simulink model "MX_Gateway"  (Simulink 9.0)
settings: Solver=FixedStepDiscrete  StartTime=0.0  StopTime=100  FixedStep=0.01  RelTol=1e-3
values from neighbouring files (symbols this model uses):
  ACTIVE = Bobcat.Parameter; CreateDefine( ACTIVE                         ,1                          ,'Define' ,'defines.h' )    [defines.m]
  ACTIVE_MODE_ONE = Bobcat.Parameter; CreateDefine( ACTIVE_MODE_ONE                ,19                         ,'Define' ,'defines.h' )    [defines.m]
  AUTHENTICATED = Bobcat.Parameter; CreateDefine( AUTHENTICATED                  ,1                          ,'Define' ,'defines.h' )    [defines.m]
  AUX_EMEA_CONTROLS = Bobcat.Parameter; CreateDefine( AUX_EMEA_CONTROLS  ,1   ,'Define' ,'defines.h' )    [enums.m]
  AUX_FLOW_HIGH = Bobcat.Parameter; CreateDefine( AUX_FLOW_HIGH           ,4  ,'Define' ,'defines.h' )    [enums.m]
  AUX_FLOW_INACTIVE = Bobcat.Parameter; CreateDefine( AUX_FLOW_INACTIVE       ,0  ,'Define' ,'defines.h' )    [enums.m]
  AUX_FLOW_LOW = Bobcat.Parameter; CreateDefine( AUX_FLOW_LOW            ,1  ,'Define' ,'defines.h' )    [enums.m]
  AUX_FLOW_MEDIUM = Bobcat.Parameter; CreateDefine( AUX_FLOW_MEDIUM         ,2  ,'Define' ,'defines.h' )    [enums.m]
  AUX_FLOW_STANDARD = Bobcat.Parameter; CreateDefine( AUX_FLOW_STANDARD       ,3  ,'Define' ,'defines.h' )    [enums.m]
  AUX_NON_FUNCTIONAL = Bobcat.Parameter; CreateDefine( AUX_NON_FUNCTIONAL ,255 ,'Define' ,'defines.h' )    [enums.m]
  BASE_ACTIVE = Bobcat.Parameter; CreateDefine( BASE_ACTIVE                    ,253                        ,'Define' ,'defines.h' )    [defines.m]
  BASE_DETENT = Bobcat.Parameter; CreateDefine( BASE_DETENT                    ,1                          ,'Define' ,'defines.h' )    [defines.m]
  BEEP_NONE = Bobcat.Parameter; CreateDefine( BEEP_NONE                      ,255                        ,'Define' ,'defines.h' )    [defines.m]
  CD_AIR_FILTER_DEBOUNCE_NORMAL = Bobcat.Parameter; CreateAliasCD( CD_AIR_FILTER_DEBOUNCE_NORMAL                        ,uint16(500)                ,'ConstantDataPointer' ,'CONSTANT_DATA' ,'AIR_FILTER_DEBOUNCE_NORMAL')    [cdata.m]    (also in hc12_cdata.m; m4_cdata.m)
  CD_AIR_FILTER_DEBOUNCE_NOT_CONNECTED = Bobcat.Parameter; CreateAliasCD( CD_AIR_FILTER_DEBOUNCE_NOT_CONNECTED                 ,uint16(3000)               ,'ConstantDataPointer' ,'CONSTANT_DATA' ,'AIR_FILTER_DEBOUNCE_NOT_CONNECTED')    [cdata.m]    (also in hc12_cdata.m; m4_cdata.m)
  CD_AIR_FILTER_DEBOUNCE_PLUGGED = Bobcat.Parameter; CreateAliasCD( CD_AIR_FILTER_DEBOUNCE_PLUGGED                       ,uint16(3000)               ,'ConstantDataPointer' ,'CONSTANT_DATA' ,'AIR_FILTER_DEBOUNCE_PLUGGED')    [cdata.m]    (also in hc12_cdata.m; m4_cdata.m)
  CD_AIR_FILTER_FUNCTION = Bobcat.Parameter; CreateAliasCD( CD_AIR_FILTER_FUNCTION                               ,uint8(255)                 ,'ConstantDataPointer' ,'CONSTANT_DATA' ,'AIR_FILTER_FUNCTION')    [cdata.m]    (also in hc12_cdata.m; m4_cdata.m)
  CD_AIR_FILTER_PLUGGED_WEIGHT = Bobcat.Parameter; CreateAliasCD( CD_AIR_FILTER_PLUGGED_WEIGHT                         ,uint8(2)                   ,'ConstantDataPointer' ,'CONSTANT_DATA' ,'AIR_FILTER_PLUGGED_WEIGHT')    [cdata.m]    (also in hc12_cdata.m; m4_cdata.m)
  CD_AIR_FILTER_UNPLUGGED_WEIGHT = Bobcat.Parameter; CreateAliasCD( CD_AIR_FILTER_UNPLUGGED_WEIGHT                       ,uint8(1)                   ,'ConstantDataPointer' ,'CONSTANT_DATA' ,'AIR_FILTER_UNPLUGGED_WEIGHT')    [cdata.m]    (also in hc12_cdata.m; m4_cdata.m)
  CD_AUTOIDLE_ARMBAR_DELAY = Bobcat.Parameter; CreateAliasCD( CD_AUTOIDLE_ARMBAR_DELAY                             ,uint8(2)                   ,'ConstantDataPointer' ,'CONSTANT_DATA' ,'AUTOIDLE_ARMBAR_DELAY')    [cdata.m]    (also in hc12_cdata.m; m4_cdata.m)
  CD_AUTOIDLE_FUNCTION = Bobcat.Parameter; CreateAliasCD( CD_AUTOIDLE_FUNCTION                                 ,uint8(255)                 ,'ConstantDataPointer' ,'CONSTANT_DATA' ,'AUTOIDLE_FUNCTION')    [cdata.m]    (also in hc12_cdata.m; m4_cdata.m)
  CD_AUTOIDLE_IDLE_DELAY = Bobcat.Parameter; CreateAliasCD( CD_AUTOIDLE_IDLE_DELAY                               ,uint8(40)                  ,'ConstantDataPointer' ,'CONSTANT_DATA' ,'AUTOIDLE_IDLE_DELAY')    [cdata.m]    (also in hc12_cdata.m; m4_cdata.m)
  CD_AUTOIDLE_LOW_IDLE_START = Bobcat.Parameter; CreateAliasCD( CD_AUTOIDLE_LOW_IDLE_START                           ,uint8(255)                 ,'ConstantDataPointer' ,'CONSTANT_DATA' ,'AUTOIDLE_LOW_IDLE_START')    [cdata.m]    (also in hc12_cdata.m; m4_cdata.m)
  CD_AUTOIDLE_PRESSURE_OFF = Bobcat.Parameter; CreateAliasCD( CD_AUTOIDLE_PRESSURE_OFF                             ,uint16(300)                ,'ConstantDataPointer' ,'CONSTANT_DATA' ,'AUTOIDLE_PRESSURE_OFF')    [cdata.m]    (also in hc12_cdata.m; m4_cdata.m)
  CD_AUTOIDLE_PRESSURE_ON = Bobcat.Parameter; CreateAliasCD( CD_AUTOIDLE_PRESSURE_ON                              ,uint16(270)                ,'ConstantDataPointer' ,'CONSTANT_DATA' ,'AUTOIDLE_PRESSURE_ON')    [cdata.m]    (also in hc12_cdata.m; m4_cdata.m)
  CD_AUTOSHIFT_FUNCTION = Bobcat.Parameter; CreateAliasCD( CD_AUTOSHIFT_FUNCTION                                ,uint8(255)                 ,'ConstantDataPointer' ,'CONSTANT_DATA' ,'AUTOSHIFT_FUNCTION')    [cdata.m]    (also in hc12_cdata.m; m4_cdata.m)
  CD_AUTOSHIFT_PHIGH1 = Bobcat.Parameter; CreateAliasCD( CD_AUTOSHIFT_PHIGH1                                  ,uint16(205)                ,'ConstantDataPointer' ,'CONSTANT_DATA' ,'AUTOSHIFT_PHIGH1')    [cdata.m]    (also in hc12_cdata.m; m4_cdata.m)
  CD_AUTOSHIFT_PHIGH2 = Bobcat.Parameter; CreateAliasCD( CD_AUTOSHIFT_PHIGH2                                  ,uint16(193)                ,'ConstantDataPointer' ,'CONSTANT_DATA' ,'AUTOSHIFT_PHIGH2')    [cdata.m]    (also in hc12_cdata.m; m4_cdata.m)
  CD_AUTOSHIFT_PHIGH_TEMP1 = Bobcat.Parameter; CreateAliasCD( CD_AUTOSHIFT_PHIGH_TEMP1                             ,int16(70)                  ,'ConstantDataPointer' ,'CONSTANT_DATA' ,'AUTOSHIFT_PHIGH_TEMP1')    [cdata.m]    (also in hc12_cdata.m; m4_cdata.m)
  CD_AUTOSHIFT_PHIGH_TEMP2 = Bobcat.Parameter; CreateAliasCD( CD_AUTOSHIFT_PHIGH_TEMP2                             ,int16(160)                 ,'ConstantDataPointer' ,'CONSTANT_DATA' ,'AUTOSHIFT_PHIGH_TEMP2')    [cdata.m]    (also in hc12_cdata.m; m4_cdata.m)
  CD_AUTOSHIFT_PLOW1 = Bobcat.Parameter; CreateAliasCD( CD_AUTOSHIFT_PLOW1                                   ,uint16(130)                ,'ConstantDataPointer' ,'CONSTANT_DATA' ,'AUTOSHIFT_PLOW1')    [cdata.m]    (also in hc12_cdata.m; m4_cdata.m)
  CD_AUTOSHIFT_PLOW2 = Bobcat.Parameter; CreateAliasCD( CD_AUTOSHIFT_PLOW2                                   ,uint16(100)                ,'ConstantDataPointer' ,'CONSTANT_DATA' ,'AUTOSHIFT_PLOW2')    [cdata.m]    (also in hc12_cdata.m; m4_cdata.m)
  CD_AUTOSHIFT_PLOW_TEMP1 = Bobcat.Parameter; CreateAliasCD( CD_AUTOSHIFT_PLOW_TEMP1                              ,int16(20)                  ,'ConstantDataPointer' ,'CONSTANT_DATA' ,'AUTOSHIFT_PLOW_TEMP1')    [cdata.m]    (also in hc12_cdata.m; m4_cdata.m)
  CD_AUTOSHIFT_PLOW_TEMP2 = Bobcat.Parameter; CreateAliasCD( CD_AUTOSHIFT_PLOW_TEMP2                              ,int16(80)                  ,'ConstantDataPointer' ,'CONSTANT_DATA' ,'AUTOSHIFT_PLOW_TEMP2')    [cdata.m]    (also in hc12_cdata.m; m4_cdata.m)
  CD_AUTOSHIFT_TRAVEL_DELAY = Bobcat.Parameter; CreateAliasCD( CD_AUTOSHIFT_TRAVEL_DELAY                            ,uint8(32)                  ,'ConstantDataPointer' ,'CONSTANT_DATA' ,'AUTOSHIFT_TRAVEL_DELAY')    [cdata.m]    (also in hc12_cdata.m; m4_cdata.m)
  CD_AUX2_CRACKPOINT_CURRENT = Bobcat.Parameter; CreateAliasCD( CD_AUX2_CRACKPOINT_CURRENT                           ,fi(0,0,16,12,'hex','0c28') ,'ConstantDataPointer' ,'CONSTANT_DATA' ,'AUX2_CRACKPOINT_CURRENT')    [cdata.m]    (also in hc12_cdata.m; m4_cdata.m)
  CD_AUX2_FLOW_LOW_FUNCTION = Bobcat.Parameter; CreateAliasCD( CD_AUX2_FLOW_LOW_FUNCTION                            ,uint8(255)                 ,'ConstantDataPointer' ,'CONSTANT_DATA' ,'AUX2_FLOW_LOW_FUNCTION')    [cdata.m]    (also in hc12_cdata.m; m4_cdata.m)
  CD_AUX2_FLOW_MED_FUNCTION = Bobcat.Parameter; CreateAliasCD( CD_AUX2_FLOW_MED_FUNCTION                            ,uint8(255)                 ,'ConstantDataPointer' ,'CONSTANT_DATA' ,'AUX2_FLOW_MED_FUNCTION')    [cdata.m]    (also in hc12_cdata.m; m4_cdata.m)
  CD_AUX2_HI_FLOW_PERCENT = Bobcat.Parameter; CreateAliasCD( CD_AUX2_HI_FLOW_PERCENT                              ,fi(0,0,16,16,'hex','ffff') ,'ConstantDataPointer' ,'CONSTANT_DATA' ,'AUX2_HI_FLOW_PERCENT')    [cdata.m]    (also in hc12_cdata.m; m4_cdata.m)
  CD_AUX2_LOW_FLOW_PERCENT = Bobcat.Parameter; CreateAliasCD( CD_AUX2_LOW_FLOW_PERCENT                             ,fi(0,0,16,16,'hex','7333') ,'ConstantDataPointer' ,'CONSTANT_DATA' ,'AUX2_LOW_FLOW_PERCENT')    [cdata.m]    (also in hc12_cdata.m; m4_cdata.m)
  CD_AUX2_MAX_FLOW_CURRENT = Bobcat.Parameter; CreateAliasCD( CD_AUX2_MAX_FLOW_CURRENT                             ,fi(0,0,16,12,'hex','14cc') ,'ConstantDataPointer' ,'CONSTANT_DATA' ,'AUX2_MAX_FLOW_CURRENT')    [cdata.m]    (also in hc12_cdata.m; m4_cdata.m)
  CD_AUX2_MED_FLOW_PERCENT = Bobcat.Parameter; CreateAliasCD( CD_AUX2_MED_FLOW_PERCENT                             ,fi(0,0,16,16,'hex','9999') ,'ConstantDataPointer' ,'CONSTANT_DATA' ,'AUX2_MED_FLOW_PERCENT')    [cdata.m]    (also in hc12_cdata.m; m4_cdata.m)
  CD_AUX_CRACKPOINT_CURRENT = Bobcat.Parameter; CreateAliasCD( CD_AUX_CRACKPOINT_CURRENT                            ,fi(0,0,16,12,'hex','0000') ,'ConstantDataPointer' ,'CONSTANT_DATA' ,'AUX_CRACKPOINT_CURRENT')    [cdata.m]    (also in hc12_cdata.m; m4_cdata.m)
  CD_AUX_DETENT_FLASH_TIME = Bobcat.Parameter; CreateAliasCD( CD_AUX_DETENT_FLASH_TIME                             ,uint16(72)                 ,'ConstantDataPointer' ,'CONSTANT_DATA' ,'AUX_DETENT_FLASH_TIME')    [cdata.m]    (also in hc12_cdata.m; m4_cdata.m)
  CD_AUX_DETENT_HOLD_TIME = Bobcat.Parameter; CreateAliasCD( CD_AUX_DETENT_HOLD_TIME                              ,uint16(100)                ,'ConstantDataPointer' ,'CONSTANT_DATA' ,'AUX_DETENT_HOLD_TIME')    [cdata.m]    (also in hc12_cdata.m; m4_cdata.m)
  CD_AUX_FULL_ON_AUX1 = Bobcat.Parameter; CreateAliasCD( CD_AUX_FULL_ON_AUX1                                  ,fi(0,0,16,12,'hex','10cd') ,'ConstantDataPointer' ,'CONSTANT_DATA' ,'AUX_FULL_ON_AUX1')    [cdata.m]    (also in hc12_cdata.m; m4_cdata.m)
  CD_AUX_FULL_ON_AUX2 = Bobcat.Parameter; CreateAliasCD( CD_AUX_FULL_ON_AUX2                                  ,fi(0,0,16,12,'hex','139d') ,'ConstantDataPointer' ,'CONSTANT_DATA' ,'AUX_FULL_ON_AUX2')    [cdata.m]    (also in hc12_cdata.m; m4_cdata.m)
  CD_AUX_FULL_ON_AUX3 = Bobcat.Parameter; CreateAliasCD( CD_AUX_FULL_ON_AUX3                                  ,fi(0,0,16,12,'hex','17d7') ,'ConstantDataPointer' ,'CONSTANT_DATA' ,'AUX_FULL_ON_AUX3')    [cdata.m]    (also in hc12_cdata.m; m4_cdata.m)
  CD_AUX_FUNCTION = Bobcat.Parameter; CreateAliasCD( CD_AUX_FUNCTION                                      ,uint8(0)                   ,'ConstantDataPointer' ,'CONSTANT_DATA' ,'AUX_FUNCTION')    [cdata.m]    (also in hc12_cdata.m; m4_cdata.m)
  CD_AUX_RATE_LIMIT_CRACKPOINT = Bobcat.Parameter; CreateAliasCD( CD_AUX_RATE_LIMIT_CRACKPOINT                         ,fi(0,0,16,12,'hex','0800') ,'ConstantDataPointer' ,'CONSTANT_DATA' ,'AUX_RATE_LIMIT_CRACKPOINT')    [cdata.m]    (also in hc12_cdata.m; m4_cdata.m)
  CD_AUX_RATE_LIMIT_CRACKPOINT_RATE = Bobcat.Parameter; CreateAliasCD( CD_AUX_RATE_LIMIT_CRACKPOINT_RATE                    ,fi(0,1,32,12,'int',102400) ,'ConstantDataPointer' ,'CONSTANT_DATA' ,'AUX_RATE_LIMIT_CRACKPOINT_RATE')    [cdata.m]    (also in hc12_cdata.m; m4_cdata.m)
  CD_AUX_RATE_LIMIT_FUNCTION = Bobcat.Parameter; CreateAliasCD( CD_AUX_RATE_LIMIT_FUNCTION                           ,uint8(255)                 ,'ConstantDataPointer' ,'CONSTANT_DATA' ,'AUX_RATE_LIMIT_FUNCTION')    [cdata.m]    (also in hc12_cdata.m; m4_cdata.m)
  CD_AUX_RATE_LIMIT_MAX_ENG_SPD = Bobcat.Parameter; CreateAliasCD( CD_AUX_RATE_LIMIT_MAX_ENG_SPD                        ,uint16(1600)               ,'ConstantDataPointer' ,'CONSTANT_DATA' ,'AUX_RATE_LIMIT_MAX_ENG_SPD')    [cdata.m]    (also in hc12_cdata.m; m4_cdata.m)
  CD_AUX_RATE_LIMIT_MIN_ENG_SPD = Bobcat.Parameter; CreateAliasCD( CD_AUX_RATE_LIMIT_MIN_ENG_SPD                        ,uint16(100)                ,'ConstantDataPointer' ,'CONSTANT_DATA' ,'AUX_RATE_LIMIT_MIN_ENG_SPD')    [cdata.m]    (also in hc12_cdata.m; m4_cdata.m)
  CD_AUX_RATE_LIMIT_NORMAL_RATE = Bobcat.Parameter; CreateAliasCD( CD_AUX_RATE_LIMIT_NORMAL_RATE                        ,fi(0,1,32,12,'int',2048)   ,'ConstantDataPointer' ,'CONSTANT_DATA' ,'AUX_RATE_LIMIT_NORMAL_RATE')    [cdata.m]    (also in hc12_cdata.m; m4_cdata.m)
  CD_AUXPOT_CAL_DEADBAND_HIGH = Bobcat.Parameter; CreateAliasCD( CD_AUXPOT_CAL_DEADBAND_HIGH                          ,uint16(574)                ,'ConstantDataPointer' ,'CONSTANT_DATA' ,'AUXPOT_CAL_DEADBAND_HIGH')    [cdata.m]    (also in hc12_cdata.m; m4_cdata.m)
  CD_AUXPOT_CAL_DEADBAND_LOW = Bobcat.Parameter; CreateAliasCD( CD_AUXPOT_CAL_DEADBAND_LOW                           ,uint16(424)                ,'ConstantDataPointer' ,'CONSTANT_DATA' ,'AUXPOT_CAL_DEADBAND_LOW')    [cdata.m]    (also in hc12_cdata.m; m4_cdata.m)
  CD_AUXPOT_CENTER_RATIO = Bobcat.Parameter; CreateAliasCD( CD_AUXPOT_CENTER_RATIO                               ,uint16(500)                ,'ConstantDataPointer' ,'CONSTANT_DATA' ,'AUXPOT_CENTER_RATIO')    [cdata.m]    (also in hc12_cdata.m; m4_cdata.m)
  CD_AUXPOT_DEADBAND = Bobcat.Parameter; CreateAliasCD( CD_AUXPOT_DEADBAND                                   ,uint8(38)                  ,'ConstantDataPointer' ,'CONSTANT_DATA' ,'AUXPOT_DEADBAND')    [cdata.m]    (also in hc12_cdata.m; m4_cdata.m)
  CD_AUXPOT_FUNCTION = Bobcat.Parameter; CreateAliasCD( CD_AUXPOT_FUNCTION                                   ,uint8(0)                   ,'ConstantDataPointer' ,'CONSTANT_DATA' ,'AUXPOT_FUNCTION')    [cdata.m]    (also in hc12_cdata.m; m4_cdata.m)
  CD_AUXPOT_RATIO_GAIN = Bobcat.Parameter; CreateAliasCD( CD_AUXPOT_RATIO_GAIN                                 ,uint16(1000)               ,'ConstantDataPointer' ,'CONSTANT_DATA' ,'AUXPOT_RATIO_GAIN')    [cdata.m]    (also in hc12_cdata.m; m4_cdata.m)
  CD_CAN_THROTTLE_FUNCTION = Bobcat.Parameter; CreateAliasCD( CD_CAN_THROTTLE_FUNCTION                             ,uint8(255)                 ,'ConstantDataPointer' ,'CONSTANT_DATA' ,'CAN_THROTTLE_FUNCTION')    [cdata.m]    (also in hc12_cdata.m; m4_cdata.m)
  CD_CAN_THROTTLE_REVERSE = Bobcat.Parameter; CreateAliasCD( CD_CAN_THROTTLE_REVERSE                              ,uint8(255)                 ,'ConstantDataPointer' ,'CONSTANT_DATA' ,'CAN_THROTTLE_REVERSE')    [cdata.m]    (also in hc12_cdata.m; m4_cdata.m)
  CD_CONSOLE_DEBOUNCE_ABSENT = Bobcat.Parameter; CreateAliasCD( CD_CONSOLE_DEBOUNCE_ABSENT                           ,uint16(12)                 ,'ConstantDataPointer' ,'CONSTANT_DATA' ,'CONSOLE_DEBOUNCE_ABSENT')    [cdata.m]    (also in hc12_cdata.m; m4_cdata.m)
  CD_CONSOLE_DEBOUNCE_OORH = Bobcat.Parameter; CreateAliasCD( CD_CONSOLE_DEBOUNCE_OORH                             ,uint16(12)                 ,'ConstantDataPointer' ,'CONSTANT_DATA' ,'CONSOLE_DEBOUNCE_OORH')    [cdata.m]    (also in hc12_cdata.m; m4_cdata.m)
  CD_CONSOLE_DEBOUNCE_OORL = Bobcat.Parameter; CreateAliasCD( CD_CONSOLE_DEBOUNCE_OORL                             ,uint16(12)                 ,'ConstantDataPointer' ,'CONSTANT_DATA' ,'CONSOLE_DEBOUNCE_OORL')    [cdata.m]    (also in hc12_cdata.m; m4_cdata.m)
  CD_CONSOLE_DEBOUNCE_PRESENT = Bobcat.Parameter; CreateAliasCD( CD_CONSOLE_DEBOUNCE_PRESENT                          ,uint16(12)                 ,'ConstantDataPointer' ,'CONSTANT_DATA' ,'CONSOLE_DEBOUNCE_PRESENT')    [cdata.m]    (also in hc12_cdata.m; m4_cdata.m)
  CD_CONSOLE_FUNCTION = Bobcat.Parameter; CreateAliasCD( CD_CONSOLE_FUNCTION                                  ,uint8(0)                   ,'ConstantDataPointer' ,'CONSTANT_DATA' ,'CONSOLE_FUNCTION')    [cdata.m]    (also in hc12_cdata.m; m4_cdata.m)
  CD_CONSOLE_OORH = Bobcat.Parameter; CreateAliasCD( CD_CONSOLE_OORH                                      ,uint16(740)                ,'ConstantDataPointer' ,'CONSTANT_DATA' ,'CONSOLE_OORH')    [cdata.m]    (also in hc12_cdata.m; m4_cdata.m)
  CD_CONSOLE_OORL = Bobcat.Parameter; CreateAliasCD( CD_CONSOLE_OORL                                      ,uint16(10)                 ,'ConstantDataPointer' ,'CONSTANT_DATA' ,'CONSOLE_OORL')    [cdata.m]    (also in hc12_cdata.m; m4_cdata.m)
  CD_CONSOLE_PRESENT_HIGH = Bobcat.Parameter; CreateAliasCD( CD_CONSOLE_PRESENT_HIGH                              ,uint16(515)                ,'ConstantDataPointer' ,'CONSTANT_DATA' ,'CONSOLE_PRESENT_HIGH')    [cdata.m]    (also in hc12_cdata.m; m4_cdata.m)
  CD_CONSOLE_PRESENT_LOW = Bobcat.Parameter; CreateAliasCD( CD_CONSOLE_PRESENT_LOW                               ,uint16(375)                ,'ConstantDataPointer' ,'CONSTANT_DATA' ,'CONSOLE_PRESENT_LOW')    [cdata.m]    (also in hc12_cdata.m; m4_cdata.m)
  CD_DELUXE_GEN5_5_FUNCTION = Bobcat.Parameter; CreateAliasCD( CD_DELUXE_GEN5_5_FUNCTION                            ,uint8(255)                 ,'ConstantDataPointer' ,'CONSTANT_DATA' ,'DELUXE_GEN5_5_FUNCTION')    [cdata.m]    (also in hc12_cdata.m; m4_cdata.m)
  CD_DELUXE_GEN5_FUNCTION = Bobcat.Parameter; CreateAliasCD( CD_DELUXE_GEN5_FUNCTION                              ,uint8(255)                 ,'ConstantDataPointer' ,'CONSTANT_DATA' ,'DELUXE_GEN5_FUNCTION')    [cdata.m]    (also in hc12_cdata.m; m4_cdata.m)
  CD_DERATE_ENGINE_SPEED_DEBOUNCE_HIGH = Bobcat.Parameter; CreateAliasCD( CD_DERATE_ENGINE_SPEED_DEBOUNCE_HIGH                 ,uint16(200)                ,'ConstantDataPointer' ,'CONSTANT_DATA' ,'DERATE_ENGINE_SPEED_DEBOUNCE_HIGH')    [cdata.m]    (also in hc12_cdata.m; m4_cdata.m)
  CD_DERATE_ENGINE_SPEED_DEBOUNCE_SHUTDOWN = Bobcat.Parameter; CreateAliasCD( CD_DERATE_ENGINE_SPEED_DEBOUNCE_SHUTDOWN             ,uint16(1000)               ,'ConstantDataPointer' ,'CONSTANT_DATA' ,'DERATE_ENGINE_SPEED_DEBOUNCE_SHUTDOWN')    [cdata.m]    (also in hc12_cdata.m; m4_cdata.m)
  CD_DERATE_ENGINE_SPEED_HIGH_OFFSET = Bobcat.Parameter; CreateAliasCD( CD_DERATE_ENGINE_SPEED_HIGH_OFFSET                   ,uint16(200)                ,'ConstantDataPointer' ,'CONSTANT_DATA' ,'DERATE_ENGINE_SPEED_HIGH_OFFSET')    [cdata.m]    (also in hc12_cdata.m; m4_cdata.m)
  CD_DERATE_METHOD = Bobcat.Parameter; CreateAliasCD( CD_DERATE_METHOD                                     ,uint8(0)                   ,'ConstantDataPointer' ,'CONSTANT_DATA' ,'DERATE_METHOD')    [cdata.m]    (also in hc12_cdata.m; m4_cdata.m)
  CD_DUMP_VALVE_FUNCTION = Bobcat.Parameter; CreateAliasCD( CD_DUMP_VALVE_FUNCTION                               ,uint8(255)                 ,'ConstantDataPointer' ,'CONSTANT_DATA' ,'DUMP_VALVE_FUNCTION')    [cdata.m]    (also in hc12_cdata.m; m4_cdata.m)
  CD_DUMP_VALVE_ON_CURRENT = Bobcat.Parameter; CreateAliasCD( CD_DUMP_VALVE_ON_CURRENT                             ,fi(0,0,16,12,'hex','ffff') ,'ConstantDataPointer' ,'CONSTANT_DATA' ,'DUMP_VALVE_ON_CURRENT')    [cdata.m]    (also in hc12_cdata.m; m4_cdata.m)
  CD_ECO_MODE_THROTTLE_REDUCTION = Bobcat.Parameter; CreateAliasCD( CD_ECO_MODE_THROTTLE_REDUCTION                       ,uint16(850)                ,'ConstantDataPointer' ,'CONSTANT_DATA' ,'ECO_MODE_THROTTLE_REDUCTION')    [cdata.m]    (also in hc12_cdata.m; m4_cdata.m)
  CD_ECT_FILTER_GAIN = Bobcat.Parameter; CreateAliasCD( CD_ECT_FILTER_GAIN                                   ,uint8(6)                   ,'ConstantDataPointer' ,'CONSTANT_DATA' ,'ECT_FILTER_GAIN')    [cdata.m]    (also in hc12_cdata.m; m4_cdata.m)
  CD_ECU_FUNCTION = Bobcat.Parameter; CreateAliasCD( CD_ECU_FUNCTION                                      ,uint8(255)                 ,'ConstantDataPointer' ,'CONSTANT_DATA' ,'ECU_FUNCTION')    [cdata.m]    (also in hc12_cdata.m; m4_cdata.m)
  CD_ECU_HOLD_DEBOUNCE_ERROR_ON = Bobcat.Parameter; CreateAliasCD( CD_ECU_HOLD_DEBOUNCE_ERROR_ON                        ,uint16(20)                 ,'ConstantDataPointer' ,'CONSTANT_DATA' ,'ECU_HOLD_DEBOUNCE_ERROR_ON')    [cdata.m]    (also in hc12_cdata.m; m4_cdata.m)
  CD_EMEA_CONTROLS_HOLD_TIME = Bobcat.Parameter; CreateAliasCD( CD_EMEA_CONTROLS_HOLD_TIME                           ,uint16(30)                 ,'ConstantDataPointer' ,'CONSTANT_DATA' ,'EMEA_CONTROLS_HOLD_TIME')    [cdata.m]    (also in hc12_cdata.m; m4_cdata.m)
  CD_EMEA_CONTROLS_OPTION_A = Bobcat.Parameter; CreateAliasCD( CD_EMEA_CONTROLS_OPTION_A                            ,uint8(255)                 ,'ConstantDataPointer' ,'CONSTANT_DATA' ,'EMEA_CONTROLS_OPTION_A')    [cdata.m]    (also in hc12_cdata.m; m4_cdata.m)
  CD_EMEA_CONTROLS_OPTION_C = Bobcat.Parameter; CreateAliasCD( CD_EMEA_CONTROLS_OPTION_C                            ,uint8(255)                 ,'ConstantDataPointer' ,'CONSTANT_DATA' ,'EMEA_CONTROLS_OPTION_C')    [cdata.m]    (also in hc12_cdata.m; m4_cdata.m)
  CD_EMEA_CONTROLS_REVERSE = Bobcat.Parameter; CreateAliasCD( CD_EMEA_CONTROLS_REVERSE                             ,uint8(255)                 ,'ConstantDataPointer' ,'CONSTANT_DATA' ,'EMEA_CONTROLS_REVERSE')    [cdata.m]    (also in hc12_cdata.m; m4_cdata.m)
  CD_EMEA_ELECTRIC_VERSION = Bobcat.Parameter; CreateAliasCD( CD_EMEA_ELECTRIC_VERSION                             ,uint8(0)                   ,'ConstantDataPointer' ,'CONSTANT_DATA' ,'EMEA_ELECTRIC_VERSION')    [cdata.m]    (also in hc12_cdata.m; m4_cdata.m)
  CD_EMEA_POT_CAL_DEADBAND_HIGH = Bobcat.Parameter; CreateAliasCD( CD_EMEA_POT_CAL_DEADBAND_HIGH                        ,uint16(574)                ,'ConstantDataPointer' ,'CONSTANT_DATA' ,'EMEA_POT_CAL_DEADBAND_HIGH')    [cdata.m]    (also in hc12_cdata.m; m4_cdata.m)
  CD_EMEA_POT_CAL_DEADBAND_LOW = Bobcat.Parameter; CreateAliasCD( CD_EMEA_POT_CAL_DEADBAND_LOW                         ,uint16(424)                ,'ConstantDataPointer' ,'CONSTANT_DATA' ,'EMEA_POT_CAL_DEADBAND_LOW')    [cdata.m]    (also in hc12_cdata.m; m4_cdata.m)
  CD_ENGINE_RPM_FACTOR = Bobcat.Parameter; CreateAliasCD( CD_ENGINE_RPM_FACTOR                                 ,uint8(35)                  ,'ConstantDataPointer' ,'CONSTANT_DATA' ,'ENGINE_RPM_FACTOR')    [cdata.m]    (also in hc12_cdata.m; m4_cdata.m)
  CD_ENGINE_SPEED_FILTER_FAST_VALUE = Bobcat.Parameter; CreateAliasCD( CD_ENGINE_SPEED_FILTER_FAST_VALUE                    ,uint8(1)                   ,'ConstantDataPointer' ,'CONSTANT_DATA' ,'ENGINE_SPEED_FILTER_FAST_VALUE')    [cdata.m]    (also in hc12_cdata.m; m4_cdata.m)
  CD_ENGINE_SPEED_FILTER_SLOW_VALUE = Bobcat.Parameter; CreateAliasCD( CD_ENGINE_SPEED_FILTER_SLOW_VALUE                    ,uint8(4)                   ,'ConstantDataPointer' ,'CONSTANT_DATA' ,'ENGINE_SPEED_FILTER_SLOW_VALUE')    [cdata.m]    (also in hc12_cdata.m; m4_cdata.m)
  CD_ENGINE_SPEED_RUNNING = Bobcat.Parameter; CreateAliasCD( CD_ENGINE_SPEED_RUNNING                              ,uint16(800)                ,'ConstantDataPointer' ,'CONSTANT_DATA' ,'ENGINE_SPEED_RUNNING')    [cdata.m]    (also in hc12_cdata.m; m4_cdata.m)
  CD_ENGINE_START_DELAY_DEBOUNCE_RPM_NO_SIGNAL = Bobcat.Parameter; CreateAliasCD( CD_ENGINE_START_DELAY_DEBOUNCE_RPM_NO_SIGNAL         ,uint16(0)                  ,'ConstantDataPointer' ,'CONSTANT_DATA' ,'ENGINE_START_DELAY_DEBOUNCE_RPM_NO_SIGNAL')    [cdata.m]    (also in hc12_cdata.m; m4_cdata.m)
  CD_ENGINE_START_DELAY_OFF_TIME = Bobcat.Parameter; CreateAliasCD( CD_ENGINE_START_DELAY_OFF_TIME                       ,uint16(0)                  ,'ConstantDataPointer' ,'CONSTANT_DATA' ,'ENGINE_START_DELAY_OFF_TIME')    [cdata.m]    (also in hc12_cdata.m; m4_cdata.m)
  CD_ENGINE_START_DELAY_RPM_NO_SIGNAL = Bobcat.Parameter; CreateAliasCD( CD_ENGINE_START_DELAY_RPM_NO_SIGNAL                  ,uint16(0)                  ,'ConstantDataPointer' ,'CONSTANT_DATA' ,'ENGINE_START_DELAY_RPM_NO_SIGNAL')    [cdata.m]    (also in hc12_cdata.m; m4_cdata.m)
  CD_EOP_DEBOUNCE_EXTREMELY_LOW = Bobcat.Parameter; CreateAliasCD( CD_EOP_DEBOUNCE_EXTREMELY_LOW                        ,uint16(50)                 ,'ConstantDataPointer' ,'CONSTANT_DATA' ,'EOP_DEBOUNCE_EXTREMELY_LOW')    [cdata.m]    (also in hc12_cdata.m; m4_cdata.m)
  CD_EOP_DEBOUNCE_NORMAL = Bobcat.Parameter; CreateAliasCD( CD_EOP_DEBOUNCE_NORMAL                               ,uint16(50)                 ,'ConstantDataPointer' ,'CONSTANT_DATA' ,'EOP_DEBOUNCE_NORMAL')    [cdata.m]    (also in hc12_cdata.m; m4_cdata.m)
  CD_EOP_DEBOUNCE_SHUTDOWN = Bobcat.Parameter; CreateAliasCD( CD_EOP_DEBOUNCE_SHUTDOWN                             ,uint16(100)                ,'ConstantDataPointer' ,'CONSTANT_DATA' ,'EOP_DEBOUNCE_SHUTDOWN')    [cdata.m]    (also in hc12_cdata.m; m4_cdata.m)
  CD_EOP_FILTER_VALUE = Bobcat.Parameter; CreateAliasCD( CD_EOP_FILTER_VALUE                                  ,uint8(3)                   ,'ConstantDataPointer' ,'CONSTANT_DATA' ,'EOP_FILTER_VALUE')    [cdata.m]    (also in hc12_cdata.m; m4_cdata.m)
  CD_EOP_FUNCTION = Bobcat.Parameter; CreateAliasCD( CD_EOP_FUNCTION                                      ,uint8(1)                   ,'ConstantDataPointer' ,'CONSTANT_DATA' ,'EOP_FUNCTION')    [cdata.m]    (also in hc12_cdata.m; m4_cdata.m)
  CD_EOP_SWITCH_GAUGE_VALUE = Bobcat.Parameter; CreateAliasCD( CD_EOP_SWITCH_GAUGE_VALUE                            ,uint16(125)                ,'ConstantDataPointer' ,'CONSTANT_DATA' ,'EOP_SWITCH_GAUGE_VALUE')    [cdata.m]    (also in hc12_cdata.m; m4_cdata.m)
  CD_EOP_SWITCH_LOW = Bobcat.Parameter; CreateAliasCD( CD_EOP_SWITCH_LOW                                    ,uint16(50)                 ,'ConstantDataPointer' ,'CONSTANT_DATA' ,'EOP_SWITCH_LOW')    [cdata.m]    (also in hc12_cdata.m; m4_cdata.m)
  CD_EOP_SWITCH_PRESSURE_VALUE = Bobcat.Parameter; CreateAliasCD( CD_EOP_SWITCH_PRESSURE_VALUE                         ,uint16(40)                 ,'ConstantDataPointer' ,'CONSTANT_DATA' ,'EOP_SWITCH_PRESSURE_VALUE')    [cdata.m]    (also in hc12_cdata.m; m4_cdata.m)
  CD_EOT_DEBOUNCE_EXTREMELY_LOW = Bobcat.Parameter; CreateAliasCD( CD_EOT_DEBOUNCE_EXTREMELY_LOW                        ,uint16(2)                  ,'ConstantDataPointer' ,'CONSTANT_DATA' ,'EOT_DEBOUNCE_EXTREMELY_LOW')    [cdata.m]    (also in hc12_cdata.m; m4_cdata.m)
  CD_EOT_DEBOUNCE_LOW = Bobcat.Parameter; CreateAliasCD( CD_EOT_DEBOUNCE_LOW                                  ,uint16(2)                  ,'ConstantDataPointer' ,'CONSTANT_DATA' ,'EOT_DEBOUNCE_LOW')    [cdata.m]    (also in hc12_cdata.m; m4_cdata.m)
  CD_EOT_DEBOUNCE_NORMAL = Bobcat.Parameter; CreateAliasCD( CD_EOT_DEBOUNCE_NORMAL                               ,uint16(2)                  ,'ConstantDataPointer' ,'CONSTANT_DATA' ,'EOT_DEBOUNCE_NORMAL')    [cdata.m]    (also in hc12_cdata.m; m4_cdata.m)
  CD_EOT_EXTREMELY_LOW = Bobcat.Parameter; CreateAliasCD( CD_EOT_EXTREMELY_LOW                                 ,uint16(81)                 ,'ConstantDataPointer' ,'CONSTANT_DATA' ,'EOT_EXTREMELY_LOW')    [cdata.m]    (also in hc12_cdata.m; m4_cdata.m)
  CD_EOT_FUNCTION = Bobcat.Parameter; CreateAliasCD( CD_EOT_FUNCTION                                      ,uint8(255)                 ,'ConstantDataPointer' ,'CONSTANT_DATA' ,'EOT_FUNCTION')    [cdata.m]    (also in hc12_cdata.m; m4_cdata.m)
  CD_EOT_LOW = Bobcat.Parameter; CreateAliasCD( CD_EOT_LOW                                           ,uint16(90)                 ,'ConstantDataPointer' ,'CONSTANT_DATA' ,'EOT_LOW')    [cdata.m]    (also in hc12_cdata.m; m4_cdata.m)
  CD_EOT_TEMPERATURE_HYSTERESIS = Bobcat.Parameter; CreateAliasCD( CD_EOT_TEMPERATURE_HYSTERESIS                        ,uint16(2)                  ,'ConstantDataPointer' ,'CONSTANT_DATA' ,'EOT_TEMPERATURE_HYSTERESIS')    [cdata.m]    (also in hc12_cdata.m; m4_cdata.m)
  CD_FAST_TRAVEL_FUNCTION = Bobcat.Parameter; CreateAliasCD( CD_FAST_TRAVEL_FUNCTION                              ,uint8(255)                 ,'ConstantDataPointer' ,'CONSTANT_DATA' ,'FAST_TRAVEL_FUNCTION')    [cdata.m]    (also in hc12_cdata.m; m4_cdata.m)
  CD_FAST_TRAVEL_THROTTLE_DELAY = Bobcat.Parameter; CreateAliasCD( CD_FAST_TRAVEL_THROTTLE_DELAY                        ,uint16(10)                 ,'ConstantDataPointer' ,'CONSTANT_DATA' ,'FAST_TRAVEL_THROTTLE_DELAY')    [cdata.m]    (also in hc12_cdata.m; m4_cdata.m)
  CD_FAST_TRAVEL_THROTTLE_LIMIT = Bobcat.Parameter; CreateAliasCD( CD_FAST_TRAVEL_THROTTLE_LIMIT                        ,uint16(1000)               ,'ConstantDataPointer' ,'CONSTANT_DATA' ,'FAST_TRAVEL_THROTTLE_LIMIT')    [cdata.m]    (also in hc12_cdata.m; m4_cdata.m)
  CD_FLYWHEEL_TEETH = Bobcat.Parameter; CreateAliasCD( CD_FLYWHEEL_TEETH                                    ,uint8(83)                  ,'ConstantDataPointer' ,'CONSTANT_DATA' ,'FLYWHEEL_TEETH')    [cdata.m]    (also in hc12_cdata.m; m4_cdata.m)
  CD_FUEL_LEVEL_FILTER_FAST_VALUE = Bobcat.Parameter; CreateAliasCD( CD_FUEL_LEVEL_FILTER_FAST_VALUE                      ,uint8(5)                   ,'ConstantDataPointer' ,'CONSTANT_DATA' ,'FUEL_LEVEL_FILTER_FAST_VALUE')    [cdata.m]    (also in hc12_cdata.m; m4_cdata.m)
  CD_FUEL_LEVEL_FILTER_SLOW_VALUE = Bobcat.Parameter; CreateAliasCD( CD_FUEL_LEVEL_FILTER_SLOW_VALUE                      ,uint8(10)                  ,'ConstantDataPointer' ,'CONSTANT_DATA' ,'FUEL_LEVEL_FILTER_SLOW_VALUE')    [cdata.m]    (also in hc12_cdata.m; m4_cdata.m)
  CD_FUEL_PRIMARY_FEEDBACK_FUNCTION = Bobcat.Parameter; CreateAliasCD( CD_FUEL_PRIMARY_FEEDBACK_FUNCTION                    ,uint8(0)                   ,'ConstantDataPointer' ,'CONSTANT_DATA' ,'FUEL_PRIMARY_FEEDBACK_FUNCTION')    [cdata.m]    (also in hc12_cdata.m; m4_cdata.m)
  ... (+246 more)
undefined symbols (not defined in the file or in its folder): sigbuilder_block
undefined symbols (not defined in the file): CD_FUEL_RESOLUTION_TIMER
libraries: simulink x186, MX_Library x107
(part 28 of 48)

== stateflow chart MX_Gtwy_Control/Inputs/EngineSpeed/Gain ==
  state GAIN [parallel]
    settings: executionOrder=1
    state SLOW_FILTER
      du:gain_EngineSpeed = CD_ENGINE_SPEED_FILTER_SLOW_VALUE;
      SLOW_FILTER -> (junction 782) [order 1]
      (junction 782) -> (junction 783) [order 1] : [(flt_EngineSpeed > convEngineSpeed)]
      (junction 782) -> (junction 784) [order 2] : {
          | change_EngineSpeed = convEngineSpeed - flt_EngineSpeed;
          | }
      (junction 783) -> (junction 785) : {
          | change_EngineSpeed = flt_EngineSpeed - convEngineSpeed;
          | }
      (junction 785) -> (junction 784)
    state FAST_FILTER
      state CHANGE [parallel]
        settings: executionOrder=1
        CHANGE -> (junction 791)
        (junction 791) -> (junction 792) [order 1] : [(flt_EngineSpeed > convEngineSpeed)]
        (junction 791) -> (junction 793) [order 2] : {
            | change_EngineSpeed = convEngineSpeed - flt_EngineSpeed;
            | }
        (junction 792) -> (junction 794) : {
            | change_EngineSpeed = flt_EngineSpeed - convEngineSpeed;
            | }
        (junction 794) -> (junction 793)
      state FILTER [parallel]
        settings: executionOrder=2
        state FILTER_COUNT
          en:count_Factor = 0;
          du:count_Factor++;
        state FILTER
          du:gain_EngineSpeed = CD_ENGINE_SPEED_FILTER_FAST_VALUE;
        state FILTER1
          du:gain_EngineSpeed = 0;
        (default) -> FILTER_COUNT
        FILTER_COUNT -> GAIN.FAST_FILTER.FILTER.FILTER [order 1] : [(count_Factor > 1)...
            | || (convEngineSpeed < CD_ENGINE_SPEED_RUNNING)]
        FILTER1 -> FILTER_COUNT : [(convEngineSpeed != 0)]
        FILTER_COUNT -> FILTER1 [order 2] : [(convEngineSpeed == 0)]
        GAIN.FAST_FILTER.FILTER.FILTER -> FILTER1 : [(convEngineSpeed == 0)]
    (default) -> SLOW_FILTER
    FAST_FILTER -> SLOW_FILTER : [(change_EngineSpeed <= CD_ENGINE_RPM_FACTOR)...
        | && (convEngineSpeed >= CD_ENGINE_SPEED_RUNNING)]
    SLOW_FILTER -> FAST_FILTER [order 1] : [(change_EngineSpeed > CD_ENGINE_RPM_FACTOR)...
        | || (convEngineSpeed < CD_ENGINE_SPEED_RUNNING)]
  state FILTER [parallel]
    settings: executionOrder=2
    state INITIAL
      en:flt_EngineSpeed = convEngineSpeed;
    state LP_FILTER_GREATER
      du:flt_EngineSpeed = (flt_EngineSpeed + ((convEngineSpeed - flt_EngineSpeed) >> gain_EngineSpeed));
    state LP_FILTER
      du:flt_EngineSpeed = (flt_EngineSpeed - ((flt_EngineSpeed - convEngineSpeed) >> gain_EngineSpeed));
    (default) -> INITIAL
    INITIAL -> LP_FILTER [order 2]
    INITIAL -> LP_FILTER_GREATER [order 1] : [(convEngineSpeed > flt_EngineSpeed)]
    LP_FILTER -> LP_FILTER_GREATER : [(convEngineSpeed > flt_EngineSpeed)]
    LP_FILTER_GREATER -> LP_FILTER : [(convEngineSpeed <= flt_EngineSpeed)]
  data: convEngineSpeed input int16; gain_EngineSpeed output uint8; CD_ENGINE_RPM_FACTOR parameter uint8; CD_ENGINE_SPEED_FILTER_SLOW_VALUE parameter uint8; CD_ENGINE_SPEED_FILTER_FAST_VALUE parameter uint8; flt_EngineSpeed output fixdt(1,32,16); count_Factor local uint8; change_EngineSpeed local uint16; CD_ENGINE_SPEED_RUNNING parameter uint16

== matlab function MX_Gtwy_Control/PanelInterface/mod_AuxIcon/Truth_Table ==
  data: state_AuxEnable input uint8; icon_Aux output uint8; mode_RightControl input uint8; CD_AUX_FUNCTION parameter uint8; AUX_EMEA_CONTROLS parameter uint8; MODE_RIGHT_AUX1 parameter uint8
function icon_Aux = fcn(state_AuxEnable, mode_RightControl, CD_AUX_FUNCTION, AUX_EMEA_CONTROLS, MODE_RIGHT_AUX1)

aVarTruthTableCondition_1 = false;
aVarTruthTableCondition_2 = false;
aVarTruthTableCondition_3 = false;


% Aux is active

aVarTruthTableCondition_1 = logical(state_AuxEnable ~= 0);

% EMEA Controls

aVarTruthTableCondition_2 = logical(CD_AUX_FUNCTION == AUX_EMEA_CONTROLS);

% Right mode is Aux1

aVarTruthTableCondition_3 = logical(mode_RightControl == MODE_RIGHT_AUX1);

if (aVarTruthTableCondition_1 && ~aVarTruthTableCondition_2)
    aFcnTruthTableAction_1();
elseif (aVarTruthTableCondition_1 && aVarTruthTableCondition_2 && aVarTruthTableCondition_3)
    aFcnTruthTableAction_1();
else % Default
    aFcnTruthTableAction_2();
end

function aFcnTruthTableAction_1()

% Turn on icon.

icon_Aux = uint8(1);

function aFcnTruthTableAction_2()

% Turn off icon.

icon_Aux = uint8(0);

== stateflow chart MX_Gtwy_Control/Inputs/Key/Key ==
  settings: executeAtInitialization=1
  state OFF
    state DEBOUNCE
      en:debounceCount = 0;
      du:debounceCount++;
    state OFF
      en:state_Key = INACTIVE;
    (default) -> DEBOUNCE
    DEBOUNCE -> OFF.OFF : [(debounceCount >= CD_KEY_DEBOUNCE_OFF)...
        | || (state_Key == INACTIVE)]
  state ON
    en:state_Key = ACTIVE;
  state MULTIPLE
    en:state_Key = MULTIPLE;
  state Debounce
  (default) -> OFF
  MULTIPLE -> OFF : [(din_RunEnter == INACTIVE)...
      | && (din_RemoteKey == INACTIVE)]
  OFF -> ON : [(din_RunEnter == ACTIVE)...
      | || (din_RemoteKey == ACTIVE)]
  ON -> OFF [order 1] : [(din_RunEnter == INACTIVE)...
      | && (din_RemoteKey == INACTIVE)]
  Debounce -> MULTIPLE [order 2] : [after(CD_KEY_MULTIPLE_DEBOUNCE,tick)...
      |  && CD_KEYLESS_ENTRY_FUNCTION == NON_FUNCTIONAL]
  ON -> Debounce [order 2] : [(din_RunEnter == ACTIVE )...
      | && (din_RemoteKey == ACTIVE)]
  Debounce -> ON [order 1] : [(din_RemoteKey == INACTIVE)...
      | || (din_RunEnter == INACTIVE)]
  Debounce -> MULTIPLE [order 3] : [after(CD_KEYLESS_MULTIPLE_DEBOUNCE,tick)...
      |  && CD_KEYLESS_ENTRY_FUNCTION != NON_FUNCTIONAL]
  data: din_RunEnter input uint8; din_RemoteKey input uint8; state_Key output uint8; INACTIVE parameter uint8; ACTIVE parameter uint8; MULTIPLE parameter uint8; debounceCount local uint16; CD_KEY_DEBOUNCE_OFF parameter uint16; CD_KEYLESS_ENTRY_FUNCTION parameter uint8; CD_KEYLESS_MULTIPLE_DEBOUNCE parameter uint16; CD_KEY_MULTIPLE_DEBOUNCE parameter uint16; NON_FUNCTIONAL parameter uint8

== matlab function MX_Gtwy_Control/Inputs/ECT/Truth_Table ==
  data: state input uint8; gauge output uint8
function gauge = fcn(state)

aVarTruthTableCondition_1 = false;
aVarTruthTableCondition_2 = false;


% OORH

aVarTruthTableCondition_1 = logical(state == 21);

% OORL

aVarTruthTableCondition_2 = logical(state == 22);

if (aVarTruthTableCondition_1)
    aFcnTruthTableAction_3();
elseif (aVarTruthTableCondition_2)
    aFcnTruthTableAction_1();
else % Default
    aFcnTruthTableAction_2();
end

function aFcnTruthTableAction_1()

% Gauge min

gauge = uint8(1);

function aFcnTruthTableAction_2()

% Gauge current

gauge = uint8(2);

function aFcnTruthTableAction_3()

% Gauge max

gauge = uint8(3);

== stateflow chart MX_Gtwy_Control/Inputs/FuelLevel/Fuel_Level ==
  state Monitor [parallel]
    settings: executionOrder=1
    state NON_FUNCTIONAL
      en:state_FuelLevel = NORMAL;
    state OORH
      state DEBOUNCE
        en:debounceCount = 0;
        du:debounceCount++;
      state NORMAL
        en:state_FuelLevel = OORH;
      (default) -> Monitor.OORH.DEBOUNCE
      Monitor.OORH.DEBOUNCE -> Monitor.OORH.NORMAL : [(debounceCount >= CD_FUEL_LEVEL_DEBOUNCE_OORH)...
          | || (state_FuelLevel == OORH)]
    state OORL
      state DEBOUNCE
        en:debounceCount = 0;
        du:debounceCount++;
      state NORMAL
        en:state_FuelLevel = OORL;
      (default) -> Monitor.OORL.DEBOUNCE
      Monitor.OORL.DEBOUNCE -> Monitor.OORL.NORMAL : [(debounceCount >= CD_FUEL_LEVEL_DEBOUNCE_OORL)...
          | || (state_FuelLevel == OORL)]
    state NORMAL
      state NORMAL
        state DEBOUNCE
          en:debounceCount = 0;
          du:debounceCount++;
        state NORMAL
          en:state_FuelLevel = NORMAL;
        (default) -> Monitor.NORMAL.NORMAL.DEBOUNCE
        Monitor.NORMAL.NORMAL.DEBOUNCE -> Monitor.NORMAL.NORMAL.NORMAL : [(debounceCount >= CD_FUEL_LEVEL_DEBOUNCE_NORMAL)...
            | || (state_FuelLevel == NORMAL)]
      state LOW
        state DEBOUNCE
          en:debounceCount = 0;
          du:debounceCount++;
        state LOW
          en:state_FuelLevel = LOW;
        (default) -> Monitor.NORMAL.LOW.DEBOUNCE
        Monitor.NORMAL.LOW.DEBOUNCE -> Monitor.NORMAL.LOW.LOW : [(debounceCount >= CD_FUEL_LEVEL_DEBOUNCE_LOW)...
            | || (state_FuelLevel == LOW)]
      (default) -> Monitor.NORMAL.NORMAL
      Monitor.NORMAL.LOW -> Monitor.NORMAL.NORMAL : [(fltValue > CD_FUEL_RESET_VALUE)]
      Monitor.NORMAL.NORMAL -> Monitor.NORMAL.LOW : [(fltValue <= CD_FUEL_LEVEL_LOW)]
    (default) -> NON_FUNCTIONAL
    NON_FUNCTIONAL -> Monitor.NORMAL : [(CD_FUEL_LEVEL_FUNCTION != NON_FUNCTIONAL)]
    Monitor.NORMAL -> OORL [order 2] : [(rawValue <= CD_FUEL_LEVEL_OORL)]
    Monitor.NORMAL -> OORH [order 1] : [(rawValue >= CD_FUEL_LEVEL_OORH)]
    OORL -> Monitor.NORMAL : [(rawValue > CD_FUEL_LEVEL_OORL)]
    OORH -> Monitor.NORMAL : [(rawValue < CD_FUEL_LEVEL_OORH)]
  state FuelFillWarning [parallel]
    settings: executionOrder=2
    state Inactive
    state Active
      state Intermittant_Beep
        en:
        beep_Delay = (255c - fltValue)/8c;
      state Armed
        en:
        start_FuelLevel = fltValue;
        previous_Delay = 31;
        delay_Limit = 0;
      state Continuous
        en:
        state_Buzzer = CONTINUOUS_BEEP;
        ex:
        state_Buzzer = NO_BEEP;
      (default) -> Armed
      Intermittant_Beep -> (junction 910) [order 2] : [after(uint8(beep_Delay),tick)]
      Armed -> Intermittant_Beep : [fltValue >...
          | (start_FuelLevel + CD_FUEL_WARN_DELTA)]
      (junction 910) -> (junction 911) : {
          | state_Buzzer = SINGLE_SHORT_BEEP;
          | }
      (junction 911) -> (junction 912) [order 1] : [uint8(beep_Delay) == previous_Delay]
      (junction 911) -> (junction 913) [order 2] : {
          | previous_Delay = uint8(beep_Delay);
          | delay_Limit = 0;
          | }
      (junction 912) -> (junction 914) : {
          | delay_Limit+= uint8(beep_Delay);
          | }
      Intermittant_Beep -> Armed [order 3] : [delay_Limit > CD_FUEL_WARN_TIMEOUT]
      (junction 913) -> Intermittant_Beep
      (junction 914) -> (junction 913)
      Intermittant_Beep -> Continuous [order 1] : [beep_Delay == 0]
      Continuous -> Armed : [after(CD_FUEL_WARN_TIMEOUT,tick)]
    (default) -> Inactive
    Inactive -> Active : [CD_FUEL_WARN_FUNCTION != NON_FUNCTIONAL...
        | && state_Console == INACTIVE...
        | && (state_Engine == ENGINE_NOT_RUNNING || state_Engine == ENGINE_INITIALIZE)...
        | && state_FuelLevel != OORL...
        | && state_FuelLevel != OORH]
    Active -> Inactive : [state_Console != INACTIVE...
        | || (state_Engine != ENGINE_NOT_RUNNING && state_Engine != ENGINE_INITIALIZE)...
        | || state_FuelLevel == OORL...
        | || state_FuelLevel == OORH]
  data: state_Engine input uint8; state_Console input uint8; fltValue input uint16; rawValue input uint16; state_FuelLevel output uint8; CD_FUEL_LEVEL_FUNCTION parameter uint8; CD_FUEL_LEVEL_LOW parameter uint16; CD_FUEL_LEVEL_OORL parameter uint16; CD_FUEL_LEVEL_OORH parameter uint16; CD_FUEL_LEVEL_DEBOUNCE_NORMAL parameter uint16; CD_FUEL_LEVEL_DEBOUNCE_OORL parameter uint16; CD_FUEL_LEVEL_DEBOUNCE_OORH parameter uint16; CD_FUEL_LEVEL_DEBOUNCE_LOW parameter uint16; CD_FUEL_RESET_VALUE parameter uint8; debounceCount local uint16 =0; OORH parameter uint8; OORL parameter uint8; NON_FUNCTIONAL parameter uint8; NORMAL parameter uint8; LOW parameter uint8; CD_FUEL_WARN_DELTA parameter uint16; CD_FUEL_WARN_FUNCTION parameter uint8; CD_FUEL_WARN_TIMEOUT parameter uint16; ENGINE_NOT_RUNNING parameter uint8; INACTIVE parameter uint8; NO_BEEP parameter uint8; beep_Delay local uint16; delay_Limit local uint16; previous_Delay local uint16; start_FuelLevel local uint16; state_Buzzer data_store_memory double; CONTINUOUS_BEEP parameter uint8; SINGLE_SHORT_BEEP parameter uint8; ENGINE_INITIALIZE parameter uint8

== stateflow chart MX_Gtwy_Control/Inputs/FuelLevel/Gain ==
  (default) -> (junction 968)
  (junction 968) -> (junction 969) [order 1] : [(state_Console != INACTIVE)]
  (junction 968) -> (junction 970) [order 2] : {
      | Gain = CD_FUEL_LEVEL_FILTER_FAST_VALUE;
      | }
  (junction 969) -> (junction 971) : {
      | Gain = CD_FUEL_LEVEL_FILTER_SLOW_VALUE;
      | }
  (junction 971) -> (junction 970)
  data: state_Console input uint8; Gain output uint8; CD_FUEL_LEVEL_FILTER_SLOW_VALUE parameter uint8; CD_FUEL_LEVEL_FILTER_FAST_VALUE parameter uint8; INACTIVE parameter uint8

== stateflow chart MX_Gtwy_Control/PanelInterface/Aux_Ind/Aux2_Indicator ==
  state FLASH
    state On
      en:
      ind_Aux2 = ACTIVE;
    state Off
      en:
      ind_Aux2 = INACTIVE;
    (default) -> On
    On -> Off : [after(2,tick)]
    Off -> On : [after(2,tick)]
  state INACTIVE
    en:
    ind_Aux2 = INACTIVE;
  state DONT_CARE
    en:
    ind_Aux2 = DONT_CARE;
  state ACTIVE
    en:
    ind_Aux2 = ACTIVE;
  (default) -> INACTIVE
  INACTIVE -> FLASH [order 2] : [state_Detent == ERR_DETENT...
      | && ee_DeluxeG5 != ACTIVE...
      | && CD_DELUXE_GEN5_5_FUNCTION == NON_FUNCTIONAL]
  INACTIVE -> DONT_CARE [order 3] : [after(3,tick)]
  FLASH -> INACTIVE [order 1] : [after(CD_AUX_DETENT_FLASH_TIME,tick)...
      | && state_Detent != ERR_DETENT]
  INACTIVE -> ACTIVE [order 1] : [state_DetentEnable == ACTIVE]
  ACTIVE -> INACTIVE : [state_DetentEnable != ACTIVE]
  DONT_CARE -> FLASH [order 2] : [state_Detent == ERR_DETENT...
      | && ee_DeluxeG5 != ACTIVE...
      | && CD_DELUXE_GEN5_5_FUNCTION == NON_FUNCTIONAL]
  DONT_CARE -> ACTIVE [order 1] : [state_DetentEnable == ACTIVE]
  FLASH -> ACTIVE [order 2] : [state_DetentEnable == ACTIVE]
  data: state_DetentEnable input uint8; state_Detent input uint8; ind_Aux2 output uint8; ACTIVE parameter uint8; INACTIVE parameter uint8; DONT_CARE parameter uint8; ERR_DETENT parameter uint8; CD_AUX_DETENT_FLASH_TIME parameter uint16; ee_DeluxeG5 data_store_memory double; CD_DELUXE_GEN5_5_FUNCTION parameter uint8; NON_FUNCTIONAL parameter uint8

== matlab function MX_Gtwy_Control/Inputs/FuelLevel/Truth_Table ==
  data: state input uint8; gauge output uint8
function gauge = fcn(state)

aVarTruthTableCondition_1 = false;
aVarTruthTableCondition_2 = false;


% OORH

aVarTruthTableCondition_1 = logical(state == 21);

% OORL

aVarTruthTableCondition_2 = logical(state == 22);

if (aVarTruthTableCondition_1)
    aFcnTruthTableAction_3();
elseif (aVarTruthTableCondition_2)
    aFcnTruthTableAction_1();
else % Default
    aFcnTruthTableAction_2();
end

function aFcnTruthTableAction_1()

% Gauge min

gauge = uint8(1);

function aFcnTruthTableAction_2()

% Gauge current

gauge = uint8(2);

function aFcnTruthTableAction_3()

% Gauge max

gauge = uint8(3);

== stateflow chart MX_Gtwy_Control/Inputs/HydOilTemp/SwitchState/State_Switch ==
  state NORMAL
    state DEBOUNCE
      en:debounceCount = 0;
      du:debounceCount++;
    state NORMAL
      en:state_HOT = NORMAL;
    (default) -> NORMAL.DEBOUNCE
    NORMAL.DEBOUNCE -> NORMAL.NORMAL : [(debounceCount >= CD_HOT_DEBOUNCE_NORMAL)...
        | || (state_HOT == NORMAL)]
  state EXTREMELY_HIGH
    state DEBOUNCE
      en:debounceCount = 0;
      du:debounceCount++;
    state EXTREMELY_HIGH
      en:state_HOT = EXTREMELY_HIGH;
    (default) -> EXTREMELY_HIGH.DEBOUNCE
    EXTREMELY_HIGH.DEBOUNCE -> EXTREMELY_HIGH.EXTREMELY_HIGH : [(debounceCount >= CD_HOT_DEBOUNCE_EXTREMELY_HIGH)...
        | || (state_HOT== EXTREMELY_HIGH)]
  state SHUTDOWN
    state DEBOUNCE
      en:debounceCount = 0;
      du:debounceCount++;
    state SHUTDOWN
      en:state_HOT = SHUTDOWN;
    (default) -> SHUTDOWN.DEBOUNCE
    SHUTDOWN.DEBOUNCE -> SHUTDOWN.SHUTDOWN : [(debounceCount >= CD_HOT_DEBOUNCE_SHUTDOWN)]
  (default) -> NORMAL.NORMAL
  NORMAL -> EXTREMELY_HIGH : [(adin_HOT <= CD_HOT_SWITCH_EXTREMELY_HIGH)...
      | && (state_System == SYS_NORMAL)]
  EXTREMELY_HIGH -> NORMAL [order 1] : [(adin_HOT > CD_HOT_SWITCH_EXTREMELY_HIGH)...
      | || (state_System != SYS_NORMAL)]
  EXTREMELY_HIGH -> SHUTDOWN [order 2] : [(CD_SHUTDOWN_FUNCTION != NON_FUNCTIONAL)...
      | && (state_HOT == EXTREMELY_HIGH)]
  SHUTDOWN -> EXTREMELY_HIGH : [(adin_HOT > CD_HOT_SWITCH_EXTREMELY_HIGH)...
      | && (state_HOT != SHUTDOWN)]
  data: adin_HOT input uint16; state_HOT output uint8; debounceCount local uint16 =0; CD_HOT_DEBOUNCE_EXTREMELY_HIGH parameter uint16; CD_HOT_DEBOUNCE_NORMAL parameter uint16; CD_HOT_DEBOUNCE_SHUTDOWN parameter uint16; CD_SHUTDOWN_FUNCTION parameter uint8; EXTREMELY_HIGH parameter uint8; NON_FUNCTIONAL parameter uint8; SHUTDOWN parameter uint8; NORMAL parameter uint8; state_System input uint8; SYS_NORMAL parameter uint8; CD_HOT_SWITCH_EXTREMELY_HIGH parameter uint16
